simulink model "sma_corr"  (Simulink 8.0)
settings: Solver=VariableStepDiscrete  SolverMode=SingleTasking  StartTime=0.0  StopTime=1000  RelTol=1e-3
PreLoadFcn:
  | % Set this to 1 if simulating
  | simulating = 0;
  | 
  | % Overall parameters
  | n_comp = 128;
  | 
  | % Simulation
  | if (simulating)
  |     n_tvg_frames = 4;
  |     frames_per_vector = 4*8;
  |     vectors_per_wstep = 2;
  |     total_wsteps = 1; % 2^n
  |     % Laura's params
  |     x_ctr = 12;
  |     x_data_ctr = x_ctr-5;
  |     rx_addr_ctr = x_data_ctr+2;
  |     tx_addr_ctr = x_data_ctr+2;
  | else
  |     n_tvg_frames = 256;
  |     frames_per_vector = 256*8;
  |     vectors_per_wstep = 11;
  |     total_wsteps = 6; % 2^n
  |     % Laura's params
  |     x_ctr = 32;
  |     x_data_ctr = 18;
  |     rx_addr_ctr = x_data_ctr+2;
  |     tx_addr_ctr = x_data_ctr+2;
  | end
  | 
  | sb_states = repmat([ones(1, 256) zeros(1, 256)], 1, 2^(total_wsteps-1));
InitFcn:
  | % Get the XSG object to find the clock source
  | xps_xsg_blks = find_system(gcs,'FollowLinks','on','LookUnderMasks','all', 'SearchDepth',1, 'Tag','xps:xsg');
  | xsg_obj = xps_block(xps_xsg_blks{1}, {});
  | xsg_obj = xps_xsg(xsg_obj);
  | clk_src = get(xsg_obj, 'clk_src');
  | if strcmp(clk_src, 'adc0_clk')
  |     adc_brd = 'ZDOK 0';
  | elseif strcmp(clk_src, 'adc1_clk')
  |     adc_brd = 'ZDOK 1';
  | else
  |     adc_brd = '';
  | end
  | 
  | adc_clk_rate = 0;
  | % Get the appropriate ADC5g object
  | xps_adc5g_blks = find_system(gcs, 'FollowLinks', 'on', 'LookUnderMasks','all', 'SearchDepth',2, 'Tag','xps:adc5g');
  | for n = 1:length(xps_adc5g_blks)
  |     adc5g_blk = xps_adc5g_blks{n};
  |     if strcmp(get_param(adc5g_blk, 'adc_brd'), adc_brd)
  |         adc_clk_rate = 2*str2num(get_param(adc5g_blk, 'adc_clk_rate'));
  |     end
  | end
undefined symbols (not defined in the file or in its folder): Down, sfp, Up, X, xlipgetpartsetting, Z
libraries: xbsIndex_r4 x9231, casper_library_misc x178, dspsigops x32, casper_library_delays x26, xps_library x21
(part 24 of 92)

== system sma_corr/beng/sb1demodphase/patterns ==
  addr : Inport
  data_in : Inport  Port=2
  we : Inport  Port=3
  calc_add : Subsystem  mask{data_width=32, addr_width=10, sample_rate=sample_rate}
      mask prompts: data_width "data width"; addr_width "address width"; sample_rate "sample rate"
  convert_addr : xbsIndex_r4/Convert  gui_display_data_type=Fixed-point  arith_type=Unsigned  n_bits=addr_width  bin_pt=0  float_type=Single  exp_bits=8  fraction_bits=24  quantization=Truncate  overflow=Wrap  en=off  latency=0  dbl_ovrd=off  pipeline=off  block_type=convert
      settings: xl_area=[0, 0, 0, 0, 0, 0, 0]
  convert_din1 : xbsIndex_r4/Convert  gui_display_data_type=Fixed-point  arith_type=Unsigned  n_bits=32  bin_pt=0  float_type=Single  exp_bits=8  fraction_bits=24  quantization=Truncate  overflow=Wrap  en=off  latency=0  dbl_ovrd=off  pipeline=off  block_type=convert
      settings: xl_area=[0, 0, 0, 0, 0, 0, 0]
  convert_we : xbsIndex_r4/Convert  gui_display_data_type=Fixed-point  arith_type=Unsigned  n_bits=1  bin_pt=0  float_type=Single  exp_bits=8  fraction_bits=24  quantization=Truncate  overflow=Wrap  en=off  latency=0  dbl_ovrd=off  pipeline=off  block_type=convert
      settings: xl_area=[0, 0, 0, 0, 0, 0, 0]
  mem : Subsystem
  munge_in : Subsystem  AncestorBlock=casper_library_flow_control/munge  mask(munge){divisions=1, div_size=32, order=[0], arith_type_out=Unsigned, bin_pt_out=0}
      mask prompts: divisions "Number of divisions"; div_size "Division size (bits)"; order "Division packing order"; arith_type_out "Output arithmetic type"; bin_pt_out "Output binary point"
      mask help: Splits an input bus into the specified number of divisions and then recombines them in the order specified (most significant division = 0,least = number divisions-1)
      mask init:
      | munge_init(gcb, ...
      |     'divisions', divisions, ...
      |     'div_size', div_size, ...
      |     'order', order, ...
      |     'arith_type_out', arith_type_out, ...
      |     'bin_pt_out', bin_pt_out);
      settings: AttributesFormatString=|
      | split:32
      | join:0
      | Unsigned [32,0]
      mask TypeOptions arith_type_out: Unsigned | Signed  (2's comp) | Floating-point
  munge_out : Subsystem  AncestorBlock=casper_library_flow_control/munge  mask(munge){divisions=1, div_size=32, order=[0], arith_type_out=Unsigned, bin_pt_out=0}
      mask prompts: divisions "Number of divisions"; div_size "Division size (bits)"; order "Division packing order"; arith_type_out "Output arithmetic type"; bin_pt_out "Output binary point"
      mask help: Splits an input bus into the specified number of divisions and then recombines them in the order specified (most significant division = 0,least = number divisions-1)
      mask init:
      | munge_init(gcb, ...
      |     'divisions', divisions, ...
      |     'div_size', div_size, ...
      |     'order', order, ...
      |     'arith_type_out', arith_type_out, ...
      |     'bin_pt_out', bin_pt_out);
      settings: AttributesFormatString=|
      | split:32
      | join:0
      | Unsigned [32,0]
      mask TypeOptions arith_type_out: Unsigned | Signed  (2's comp) | Floating-point
  sma_corr_beng_sb1demodphase_patterns_addr : xbsIndex_r4/Gateway Out  inherit_from_input=off  hdl_port=on  timing_constraint=None  locs_specified=off  LOCs={'A5','B9','A9','C1','A3','G6','E9','A6','A7','A8','B8','B1','B3','D9','B5','B4','B6','C2'}  UseAsDAC=off  DACChannel='1'  block_type=gatewayout
      settings: infoedit="Gateway out block.  Converts Xilinx fixed point inputs into ouputs of type Simulink integer, double, or fixed point.<P><P>Hardware notes:  In hardware these blocks become top level output ports or are discarded, depending on how they are configured."  xl_area=[0, 0, 0, 0, 0, 0, 0]  sginterface={'Nallatech'=>{'xdspkit'=>{'non_memory_mapped_port'=>'uprev_Shared_BRAM_addr'}},'Xilinx'=>{'jtaghwcosim'=>{'non_memory_mapped_port'=>'uprev_Shared_BRAM_addr'},'xdspkit'=>{'non_memory_mapped_port'=>'uprev_Shared_BRAM_addr'}}}
  sma_corr_beng_sb1demodphase_patterns_data_in : xbsIndex_r4/Gateway Out  inherit_from_input=off  hdl_port=on  timing_constraint=None  locs_specified=off  LOCs={'B21','B22','A22','B23','B19','C19','A20','A21','A23','B24','A24','A25','E18','D18','C18','B18'}  UseAsDAC=off  DACChannel='1'  block_type=gatewayout
      settings: infoedit="Gateway out block.  Converts Xilinx fixed point inputs into ouputs of type Simulink integer, double, or fixed point.<P><P>Hardware notes:  In hardware these blocks become top level output ports or are discarded, depending on how they are configured."  xl_area=[0, 0, 0, 0, 0, 0, 0]  sginterface={'Nallatech'=>{'xdspkit'=>{'non_memory_mapped_port'=>'uprev_Shared_BRAM_data_in'}},'Xilinx'=>{'jtaghwcosim'=>{'non_memory_mapped_port'=>'uprev_Shared_BRAM_data_in'},'xdspkit'=>{'non_memory_mapped_port'=>'uprev_Shared_BRAM_data_in'}}}
  sma_corr_beng_sb1demodphase_patterns_data_out : xbsIndex_r4/Gateway In  gui_display_data_type=Fixed-point  arith_type=Unsigned  n_bits=32  bin_pt=0  preci_type=Single  exp_width=8  frac_width=24  quantization=Truncate  overflow=Wrap  period=sample_rate  dbl_ovrd=off  timing_constraint=None  locs_specified=off  LOCs={}  UseAsADC=off  ADCChannel='1'  inherit_from_input=off  hdl_port=on  block_type=gatewayin
      settings: xl_area=[0, 0, 0, 0, 0, 0, 0]
  sma_corr_beng_sb1demodphase_patterns_we : xbsIndex_r4/Gateway Out  inherit_from_input=off  hdl_port=on  timing_constraint=None  locs_specified=off  LOCs={'A14'}  UseAsDAC=off  DACChannel='1'  block_type=gatewayout
      settings: infoedit="Gateway out block.  Converts Xilinx fixed point inputs into ouputs of type Simulink integer, double, or fixed point.<P><P>Hardware notes:  In hardware these blocks become top level output ports or are discarded, depending on how they are configured."  xl_area=[0, 0, 0, 0, 0, 0, 0]
  data_out : Outport
  wires:
  convert_addr/1 -> sma_corr_beng_sb1demodphase_patterns_addr/1
  convert_din1/1 -> sma_corr_beng_sb1demodphase_patterns_data_in/1
  data_in/1 -> munge_in/1(din)
  calc_add/1(out) -> convert_addr/1
  sma_corr_beng_sb1demodphase_patterns_we/1 -> mem/3(In3)
  sma_corr_beng_sb1demodphase_patterns_data_in/1 -> mem/2(In2)
  sma_corr_beng_sb1demodphase_patterns_addr/1 -> mem/1(In1)
  convert_we/1 -> sma_corr_beng_sb1demodphase_patterns_we/1
  sma_corr_beng_sb1demodphase_patterns_data_out/1 -> munge_out/1(din)
  mem/1(Out1) -> sma_corr_beng_sb1demodphase_patterns_data_out/1
  addr/1 -> calc_add/1(in)
  we/1 -> convert_we/1
  munge_out/1(dout) -> data_out/1
  munge_in/1(dout) -> convert_din1/1

== system sma_corr/beng/sb1demodphase/pipeline ==
  d : Inport
  Register{0..4} (5 alike) : xbsIndex_r4/Register  init=0  rst=off  en=off  dbl_ovrd=off  block_type=register
      Register0 settings: block_version=11.4
      Register1 settings: block_version=11.4
      Register2 settings: block_version=11.4
  q : Outport
  wires:
  Register0/1 -> Register1/1
  Register1/1 -> Register2/1
  Register2/1 -> Register3/1
  Register3/1 -> Register4/1
  d/1 -> Register0/1
  Register4/1 -> q/1

== system sma_corr/beng/sb1demodphase/pipeline2 ==
  d : Inport
  Register{0..8} (9 alike) : xbsIndex_r4/Register  init=0  rst=off  en=off  dbl_ovrd=off  block_type=register
      Register0 settings: block_version=11.4
      Register1 settings: block_version=11.4
      Register2 settings: block_version=11.4
  q : Outport
  wires:
  Register0/1 -> Register1/1
  Register1/1 -> Register2/1
  Register2/1 -> Register3/1
  Register3/1 -> Register4/1
  Register4/1 -> Register5/1
  Register5/1 -> Register6/1
  Register6/1 -> Register7/1
  Register7/1 -> Register8/1
  d/1 -> Register0/1
  Register8/1 -> q/1

== system sma_corr/beng/sb1demodphase/pipeline3 ==
  d : Inport
  Register{0..8} (9 alike) : xbsIndex_r4/Register  init=0  rst=off  en=off  dbl_ovrd=off  block_type=register
      Register0 settings: block_version=11.4
      Register1 settings: block_version=11.4
      Register2 settings: block_version=11.4
  q : Outport
  wires:
  Register0/1 -> Register1/1
  Register1/1 -> Register2/1
  Register2/1 -> Register3/1
  Register3/1 -> Register4/1
  Register4/1 -> Register5/1
  Register5/1 -> Register6/1
  Register6/1 -> Register7/1
  Register7/1 -> Register8/1
  d/1 -> Register0/1
  Register8/1 -> q/1

== system sma_corr/beng/sb1demodphase/pipeline4 ==
  d : Inport
  Register{0..8} (9 alike) : xbsIndex_r4/Register  init=0  rst=off  en=off  dbl_ovrd=off  block_type=register
      Register0 settings: block_version=11.4
      Register1 settings: block_version=11.4
      Register2 settings: block_version=11.4
  q : Outport
  wires:
  Register0/1 -> Register1/1
  Register1/1 -> Register2/1
  Register2/1 -> Register3/1
  Register3/1 -> Register4/1
  Register4/1 -> Register5/1
  Register5/1 -> Register6/1
  Register6/1 -> Register7/1
  Register7/1 -> Register8/1
  d/1 -> Register0/1
  Register8/1 -> q/1

== system sma_corr/beng/sb1demodphase/pipeline5 ==
  d : Inport
  Register{0..4} (5 alike) : xbsIndex_r4/Register  init=0  rst=off  en=off  dbl_ovrd=off  block_type=register
      Register0 settings: block_version=11.4
      Register1 settings: block_version=11.4
      Register2 settings: block_version=11.4
  q : Outport
  wires:
  Register0/1 -> Register1/1
  Register1/1 -> Register2/1
  Register2/1 -> Register3/1
  Register3/1 -> Register4/1
  d/1 -> Register0/1
  Register4/1 -> q/1

== system sma_corr/beng/sb1demodphase/pipeline6 ==
  d : Inport
  Register{0..4} (5 alike) : xbsIndex_r4/Register  init=0  rst=off  en=off  dbl_ovrd=off  block_type=register
      Register0 settings: block_version=11.4
      Register1 settings: block_version=11.4
      Register2 settings: block_version=11.4
  q : Outport
  wires:
  Register0/1 -> Register1/1
  Register1/1 -> Register2/1
  Register2/1 -> Register3/1
  Register3/1 -> Register4/1
  d/1 -> Register0/1
  Register4/1 -> q/1

== system sma_corr/beng/sb1demodphase/pipeline7 ==
  d : Inport
  Register{0..4} (5 alike) : xbsIndex_r4/Register  init=0  rst=off  en=off  dbl_ovrd=off  block_type=register
      Register0 settings: block_version=11.4
      Register1 settings: block_version=11.4
      Register2 settings: block_version=11.4
  q : Outport
  wires:
  Register0/1 -> Register1/1
  Register1/1 -> Register2/1
  Register2/1 -> Register3/1
  Register3/1 -> Register4/1
  d/1 -> Register0/1
  Register4/1 -> q/1

== system sma_corr/beng/sb1demodphase/pipeline8 ==
  d : Inport
  Register{0..4} (5 alike) : xbsIndex_r4/Register  init=0  rst=off  en=off  dbl_ovrd=off  block_type=register
      Register0 settings: block_version=11.4
      Register1 settings: block_version=11.4
      Register2 settings: block_version=11.4
  q : Outport
  wires:
  Register0/1 -> Register1/1
  Register1/1 -> Register2/1
  Register2/1 -> Register3/1
  Register3/1 -> Register4/1
  d/1 -> Register0/1
  Register4/1 -> q/1

== system sma_corr/beng/sb1demodphase/scramble ==
  note: Each input a single polarization. This block rearranges data to interleave
      | the polarizations in each stream to match the expected input of subsequent
      | systems.
  X : Inport
  Y : Inport  Port=2
  sync : Inport  Port=3
  BitBasher : xbsIndex_r4/BitBasher  bitexpr=|  display_expr=off  sr_1=1  arith_type1=Unsigned  bin_pt1=0  sr_2=2  arith_type2=Unsigned  bin_pt2=0  sr_3=3  arith_type3=Unsigned  bin_pt3=0  sr_4=4  arith_type4=Unsigned  bin_pt4=0  dbl_ovrd=off  block_type=bitbasher
      | <!DOCTYPE HTML PUBLIC "-//W3C//DTD HTML 4.0//EN" "http://www.w3.org/TR/REC-html40/strict.dtd">
      | <html><head><meta name="qrichtext" content="1" /><style type="text/css">
      | p, li { white-space: pre-wrap; }
      | </style></head><body style=" font-family:'Ubuntu'; font-size:11pt; font-weight:400; font-style:normal;">
      | <p style=" margin-top:0px; margin-bottom:0px; margin-left:0px; margin-right:0px; -qt-block-indent:0; text-indent:0px;"><span style=" font-family:'MS Shell Dlg'; font-size:8pt;">Xab={X[127:96]}<br />Xcd={X[95:64]}<br />Xef={X[63:32]}<br />Xgh={X[31:0]}</span></p></body></html>
  BitBasher1 : xbsIndex_r4/BitBasher  bitexpr=|  display_expr=off  sr_1=1  arith_type1=Unsigned  bin_pt1=0  sr_2=2  arith_type2=Unsigned  bin_pt2=0  sr_3=3  arith_type3=Unsigned  bin_pt3=0  sr_4=4  arith_type4=Unsigned  bin_pt4=0  dbl_ovrd=off  block_type=bitbasher
      | <!DOCTYPE HTML PUBLIC "-//W3C//DTD HTML 4.0//EN" "http://www.w3.org/TR/REC-html40/strict.dtd">
      | <html><head><meta name="qrichtext" content="1" /><style type="text/css">
      | p, li { white-space: pre-wrap; }
      | </style></head><body style=" font-family:'Ubuntu'; font-size:11pt; font-weight:400; font-style:normal;">
      | <p style=" margin-top:0px; margin-bottom:0px; margin-left:0px; margin-right:0px; -qt-block-indent:0; text-indent:0px;"><span style=" font-family:'MS Shell Dlg'; font-size:8pt;">Yab={Y[127:96]}<br />Ycd={Y[95:64]}<br />Yef={Y[63:32]}<br />Ygh={Y[31:0]}</span></p></body></html>
  BitBasher2 : xbsIndex_r4/BitBasher  bitexpr=|  display_expr=off  sr_1=1  arith_type1=Unsigned  bin_pt1=0  sr_2=2  arith_type2=Unsigned  bin_pt2=0  sr_3=3  arith_type3=Unsigned  bin_pt3=0  sr_4=4  arith_type4=Unsigned  bin_pt4=0  dbl_ovrd=off  block_type=bitbasher
      | <!DOCTYPE HTML PUBLIC "-//W3C//DTD HTML 4.0//EN" "http://www.w3.org/TR/REC-html40/strict.dtd">
      | <html><head><meta name="qrichtext" content="1" /><style type="text/css">
      | p, li { white-space: pre-wrap; }
      | </style></head><body style=" font-family:'Ubuntu'; font-size:11pt; font-weight:400; font-style:normal;">
      | <p style=" margin-top:0px; margin-bottom:0px; margin-left:0px; margin-right:0px; -qt-block-indent:0; text-indent:0px;"><span style=" font-family:'MS Shell Dlg'; font-size:8pt;">d0={Xab[31:16],Yab[31:16],Xab[15:0],Yab[15:0],Xcd[31:16],Ycd[31:16],Xcd[15:0],Ycd[15:0]}</span></p></body></html>
  BitBasher3 : xbsIndex_r4/BitBasher  bitexpr=|  display_expr=off  sr_1=1  arith_type1=Unsigned  bin_pt1=0  sr_2=2  arith_type2=Unsigned  bin_pt2=0  sr_3=3  arith_type3=Unsigned  bin_pt3=0  sr_4=4  arith_type4=Unsigned  bin_pt4=0  dbl_ovrd=off  block_type=bitbasher
      | <!DOCTYPE HTML PUBLIC "-//W3C//DTD HTML 4.0//EN" "http://www.w3.org/TR/REC-html40/strict.dtd">
      | <html><head><meta name="qrichtext" content="1" /><style type="text/css">
      | p, li { white-space: pre-wrap; }
      | </style></head><body style=" font-family:'Ubuntu'; font-size:11pt; font-weight:400; font-style:normal;">
      | <p style=" margin-top:0px; margin-bottom:0px; margin-left:0px; margin-right:0px; -qt-block-indent:0; text-indent:0px;"><span style=" font-family:'MS Shell Dlg'; font-size:8pt;">d1={Xef[31:16],Yef[31:16],Xef[15:0],Yef[15:0],Xgh[31:16],Ygh[31:16],Xgh[15:0],Ygh[15:0]}</span></p></body></html>
  d0 : Outport
  d1 : Outport  Port=2
  sync_out : Outport  Port=3
  wires:
  BitBasher3/1 -> d1/1
  BitBasher2/1 -> d0/1
  X/1 -> BitBasher/1
  Y/1 -> BitBasher1/1
  sync/1 -> sync_out/1
  BitBasher/1 -> BitBasher2/1
  BitBasher/2 -> BitBasher2/2
  BitBasher1/1 -> BitBasher2/3
  BitBasher1/2 -> BitBasher2/4
  BitBasher/3 -> BitBasher3/1
  BitBasher/4 -> BitBasher3/2
  BitBasher1/3 -> BitBasher3/3
  BitBasher1/4 -> BitBasher3/4

== system sma_corr/beng/sb1demodphase/unscramble ==
  note: Each input contains 4+4-bit complex for pol0 and pol1 interleaved. This block
      | rearranges the data so that each polarization is in a single stream.
  d0 : Inport
  d1 : Inport  Port=2
  BitBasher : xbsIndex_r4/BitBasher  bitexpr=|  display_expr=off  sr_1=1  arith_type1=Unsigned  bin_pt1=0  sr_2=2  arith_type2=Unsigned  bin_pt2=0  sr_3=3  arith_type3=Unsigned  bin_pt3=0  sr_4=4  arith_type4=Unsigned  bin_pt4=0  dbl_ovrd=off  block_type=bitbasher
      | <!DOCTYPE HTML PUBLIC "-//W3C//DTD HTML 4.0//EN" "http://www.w3.org/TR/REC-html40/strict.dtd">
      | <html><head><meta name="qrichtext" content="1" /><style type="text/css">
      | p, li { white-space: pre-wrap; }
      | </style></head><body style=" font-family:'Ubuntu'; font-size:11pt; font-weight:400; font-style:normal;">
      | <p style=" margin-top:0px; margin-bottom:0px; margin-left:0px; margin-right:0px; -qt-block-indent:0; text-indent:0px;"><span style=" font-family:'MS Shell Dlg'; font-size:8pt;">Xabcd={i[63:56], i[47:40], i[31:24], i[15:8]}<br />Yabcd={i[55:48], i[39:32], i[23:16], i[7:0]}<br /></span></p></body></html>
  BitBasher1 : xbsIndex_r4/BitBasher  bitexpr=|  display_expr=off  sr_1=1  arith_type1=Unsigned  bin_pt1=0  sr_2=2  arith_type2=Unsigned  bin_pt2=0  sr_3=3  arith_type3=Unsigned  bin_pt3=0  sr_4=4  arith_type4=Unsigned  bin_pt4=0  dbl_ovrd=off  block_type=bitbasher
      | <!DOCTYPE HTML PUBLIC "-//W3C//DTD HTML 4.0//EN" "http://www.w3.org/TR/REC-html40/strict.dtd">
      | <html><head><meta name="qrichtext" content="1" /><style type="text/css">
      | p, li { white-space: pre-wrap; }
      | </style></head><body style=" font-family:'Ubuntu'; font-size:11pt; font-weight:400; font-style:normal;">
      | <p style=" margin-top:0px; margin-bottom:0px; margin-left:0px; margin-right:0px; -qt-block-indent:0; text-indent:0px;"><span style=" font-family:'MS Shell Dlg'; font-size:8pt;">Xefgh={i[63:56], i[47:40], i[31:24], i[15:8]}<br />Yefgh={i[55:48], i[39:32], i[23:16], i[7:0]}<br /></span></p></body></html>
  BitBasher2 : xbsIndex_r4/BitBasher  bitexpr=|  display_expr=off  sr_1=1  arith_type1=Unsigned  bin_pt1=0  sr_2=2  arith_type2=Unsigned  bin_pt2=0  sr_3=3  arith_type3=Unsigned  bin_pt3=0  sr_4=4  arith_type4=Unsigned  bin_pt4=0  dbl_ovrd=off  block_type=bitbasher
      | <!DOCTYPE HTML PUBLIC "-//W3C//DTD HTML 4.0//EN" "http://www.w3.org/TR/REC-html40/strict.dtd">
      | <html><head><meta name="qrichtext" content="1" /><style type="text/css">
      | p, li { white-space: pre-wrap; }
      | </style></head><body style=" font-family:'Ubuntu'; font-size:11pt; font-weight:400; font-style:normal;">
      | <p style=" margin-top:12px; margin-bottom:12px; margin-left:0px; margin-right:0px; -qt-block-indent:0; text-indent:0px;"><span style=" font-family:'MS Shell Dlg'; font-size:8pt;">X={Xabcd,Xefgh}</span></p></body></html>
  BitBasher3 : xbsIndex_r4/BitBasher  bitexpr=|  display_expr=off  sr_1=1  arith_type1=Unsigned  bin_pt1=0  sr_2=2  arith_type2=Unsigned  bin_pt2=0  sr_3=3  arith_type3=Unsigned  bin_pt3=0  sr_4=4  arith_type4=Unsigned  bin_pt4=0  dbl_ovrd=off  block_type=bitbasher
      | <!DOCTYPE HTML PUBLIC "-//W3C//DTD HTML 4.0//EN" "http://www.w3.org/TR/REC-html40/strict.dtd">
      | <html><head><meta name="qrichtext" content="1" /><style type="text/css">
      | p, li { white-space: pre-wrap; }
      | </style></head><body style=" font-family:'Ubuntu'; font-size:11pt; font-weight:400; font-style:normal;">
      | <p style=" margin-top:12px; margin-bottom:12px; margin-left:0px; margin-right:0px; -qt-block-indent:0; text-indent:0px;"><span style=" font-family:'MS Shell Dlg'; font-size:8pt;">Y={Yabcd,Yefgh}</span></p></body></html>
  x : Outport
  y : Outport  Port=2
  wires:
  d0/1 -> BitBasher/1
  BitBasher2/1 -> x/1
  BitBasher3/1 -> y/1
  d1/1 -> BitBasher1/1
  BitBasher1/1 -> BitBasher2/2
  BitBasher/1 -> BitBasher2/1
  BitBasher/2 -> BitBasher3/1
  BitBasher1/2 -> BitBasher3/2

== system sma_corr/beng/sum0/sum_Xab ==
  gain : Inport
  sync_in : Inport  Port=2
  in16 : Inport  Port=3
  disable : Inport  Port=4
  BitBasher2 : xbsIndex_r4/BitBasher  bitexpr=|  display_expr=off  sr_1=1  arith_type1=Unsigned  bin_pt1=0  sr_2=2  arith_type2=Unsigned  bin_pt2=0  sr_3=3  arith_type3=Unsigned  bin_pt3=0  sr_4=4  arith_type4=Unsigned  bin_pt4=0  dbl_ovrd=off  block_type=bitbasher
      | <!DOCTYPE HTML PUBLIC "-//W3C//DTD HTML 4.0//EN" "http://www.w3.org/TR/REC-html40/strict.dtd">
      | <html><head><meta name="qrichtext" content="1" /><style type="text/css">
      | p, li { white-space: pre-wrap; }
      | </style></head><body style=" font-family:'Sans'; font-size:10pt; font-weight:400; font-style:normal;">
      | <p style=" margin-top:12px; margin-bottom:12px; margin-left:0px; margin-right:0px; -qt-block-indent:0; text-indent:0px;"><span style=" font-family:'MS Shell Dlg'; font-size:8pt;">o={w,x,y,z}</span></p></body></html>
  Delay : xbsIndex_r4/Delay  rst=off  infoeditControl=Selection of Reset will increase slice count due to use of real FFs and instead of SRLs  en=off  latency=7  dbl_ovrd=off  reg_retiming=off  block_type=delay
  Delay1 : xbsIndex_r4/Delay  rst=off  infoeditControl=Selection of Reset will increase slice count due to use of real FFs and instead of SRLs  en=off  latency=7  dbl_ovrd=off  reg_retiming=off  block_type=delay
  gain_and_quantize : Subsystem
  gain_and_quantize1 : Subsystem
  gain_and_quantize2 : Subsystem
  gain_and_quantize3 : Subsystem
      settings: AttributesFormatString=latency = 7
  simd_vacc : Subsystem
  sync_out : Outport
  valid : Outport  Port=2
  sum16 : Outport  Port=3
  wires:
  gain_and_quantize/1(out4) -> BitBasher2/1
  gain/1 -> gain_and_quantize/1(g), gain_and_quantize1/1(g), gain_and_quantize2/1(g), gain_and_quantize3/1(g)
  gain_and_quantize1/1(out4) -> BitBasher2/2
  simd_vacc/3(w) -> gain_and_quantize/2(in8)
  gain_and_quantize2/1(out4) -> BitBasher2/3
  simd_vacc/6(z) -> gain_and_quantize3/2(in8)
  sync_in/1 -> simd_vacc/1(sync)
  in16/1 -> simd_vacc/2(in)
  simd_vacc/4(x) -> gain_and_quantize1/2(in8)
  simd_vacc/5(y) -> gain_and_quantize2/2(in8)
  simd_vacc/1(sync_out) -> Delay/1
  simd_vacc/2(valid) -> Delay1/1
  BitBasher2/1 -> sum16/1
  gain_and_quantize3/1(out4) -> BitBasher2/4
  Delay1/1 -> valid/1
  Delay/1 -> sync_out/1
  disable/1 -> simd_vacc/3(disable)

== system sma_corr/beng/sum0/sum_Xcd ==
  gain : Inport
  sync_in : Inport  Port=2
  in16 : Inport  Port=3
  disable : Inport  Port=4
  BitBasher2 : xbsIndex_r4/BitBasher  bitexpr=|  display_expr=off  sr_1=1  arith_type1=Unsigned  bin_pt1=0  sr_2=2  arith_type2=Unsigned  bin_pt2=0  sr_3=3  arith_type3=Unsigned  bin_pt3=0  sr_4=4  arith_type4=Unsigned  bin_pt4=0  dbl_ovrd=off  block_type=bitbasher
      | <!DOCTYPE HTML PUBLIC "-//W3C//DTD HTML 4.0//EN" "http://www.w3.org/TR/REC-html40/strict.dtd">
      | <html><head><meta name="qrichtext" content="1" /><style type="text/css">
      | p, li { white-space: pre-wrap; }
      | </style></head><body style=" font-family:'Sans'; font-size:10pt; font-weight:400; font-style:normal;">
      | <p style=" margin-top:12px; margin-bottom:12px; margin-left:0px; margin-right:0px; -qt-block-indent:0; text-indent:0px;"><span style=" font-family:'MS Shell Dlg'; font-size:8pt;">o={w,x,y,z}</span></p></body></html>
  Terminator : Terminator
  Terminator1 : Terminator
  gain_and_quantize : Subsystem
  gain_and_quantize1 : Subsystem
  gain_and_quantize2 : Subsystem
  gain_and_quantize3 : Subsystem
      settings: AttributesFormatString=latency = 7
  simd_vacc : Subsystem
  sum16 : Outport
  wires:
  gain_and_quantize/1(out4) -> BitBasher2/1
  gain/1 -> gain_and_quantize/1(g), gain_and_quantize1/1(g), gain_and_quantize2/1(g), gain_and_quantize3/1(g)
  gain_and_quantize1/1(out4) -> BitBasher2/2
  simd_vacc/3(w) -> gain_and_quantize/2(in8)
  gain_and_quantize2/1(out4) -> BitBasher2/3
  simd_vacc/6(z) -> gain_and_quantize3/2(in8)
  sync_in/1 -> simd_vacc/1(sync)
  in16/1 -> simd_vacc/2(in)
  simd_vacc/4(x) -> gain_and_quantize1/2(in8)
  simd_vacc/5(y) -> gain_and_quantize2/2(in8)
  BitBasher2/1 -> sum16/1
  gain_and_quantize3/1(out4) -> BitBasher2/4
  disable/1 -> simd_vacc/3(disable)
  simd_vacc/1(sync_out) -> Terminator/1
  simd_vacc/2(valid) -> Terminator1/1

== system sma_corr/beng/sum0/sum_Yab ==
  gain : Inport
  sync_in : Inport  Port=2
  in16 : Inport  Port=3
  disable : Inport  Port=4
  BitBasher2 : xbsIndex_r4/BitBasher  bitexpr=|  display_expr=off  sr_1=1  arith_type1=Unsigned  bin_pt1=0  sr_2=2  arith_type2=Unsigned  bin_pt2=0  sr_3=3  arith_type3=Unsigned  bin_pt3=0  sr_4=4  arith_type4=Unsigned  bin_pt4=0  dbl_ovrd=off  block_type=bitbasher
      | <!DOCTYPE HTML PUBLIC "-//W3C//DTD HTML 4.0//EN" "http://www.w3.org/TR/REC-html40/strict.dtd">
      | <html><head><meta name="qrichtext" content="1" /><style type="text/css">
      | p, li { white-space: pre-wrap; }
      | </style></head><body style=" font-family:'Sans'; font-size:10pt; font-weight:400; font-style:normal;">
      | <p style=" margin-top:12px; margin-bottom:12px; margin-left:0px; margin-right:0px; -qt-block-indent:0; text-indent:0px;"><span style=" font-family:'MS Shell Dlg'; font-size:8pt;">o={w,x,y,z}</span></p></body></html>
  Terminator : Terminator
  Terminator1 : Terminator
  gain_and_quantize : Subsystem
  gain_and_quantize1 : Subsystem
  gain_and_quantize2 : Subsystem
  gain_and_quantize3 : Subsystem
      settings: AttributesFormatString=latency = 7
  simd_vacc : Subsystem
  sum16 : Outport
  wires:
  simd_vacc/2(valid) -> Terminator1/1
  simd_vacc/1(sync_out) -> Terminator/1
  disable/1 -> simd_vacc/3(disable)
  gain_and_quantize3/1(out4) -> BitBasher2/4
  BitBasher2/1 -> sum16/1
  simd_vacc/5(y) -> gain_and_quantize2/2(in8)
  simd_vacc/4(x) -> gain_and_quantize1/2(in8)
  in16/1 -> simd_vacc/2(in)
  sync_in/1 -> simd_vacc/1(sync)
  simd_vacc/6(z) -> gain_and_quantize3/2(in8)
  gain_and_quantize2/1(out4) -> BitBasher2/3
  simd_vacc/3(w) -> gain_and_quantize/2(in8)
  gain_and_quantize1/1(out4) -> BitBasher2/2
  gain/1 -> gain_and_quantize3/1(g), gain_and_quantize2/1(g), gain_and_quantize1/1(g), gain_and_quantize/1(g)
  gain_and_quantize/1(out4) -> BitBasher2/1

== system sma_corr/beng/sum0/sum_Ycd ==
  gain : Inport
  sync_in : Inport  Port=2
  in16 : Inport  Port=3
  disable : Inport  Port=4
  BitBasher2 : xbsIndex_r4/BitBasher  bitexpr=|  display_expr=off  sr_1=1  arith_type1=Unsigned  bin_pt1=0  sr_2=2  arith_type2=Unsigned  bin_pt2=0  sr_3=3  arith_type3=Unsigned  bin_pt3=0  sr_4=4  arith_type4=Unsigned  bin_pt4=0  dbl_ovrd=off  block_type=bitbasher
      | <!DOCTYPE HTML PUBLIC "-//W3C//DTD HTML 4.0//EN" "http://www.w3.org/TR/REC-html40/strict.dtd">
      | <html><head><meta name="qrichtext" content="1" /><style type="text/css">
      | p, li { white-space: pre-wrap; }
      | </style></head><body style=" font-family:'Sans'; font-size:10pt; font-weight:400; font-style:normal;">
      | <p style=" margin-top:12px; margin-bottom:12px; margin-left:0px; margin-right:0px; -qt-block-indent:0; text-indent:0px;"><span style=" font-family:'MS Shell Dlg'; font-size:8pt;">o={w,x,y,z}</span></p></body></html>
  Terminator : Terminator
  Terminator1 : Terminator
  gain_and_quantize : Subsystem
  gain_and_quantize1 : Subsystem
  gain_and_quantize2 : Subsystem
  gain_and_quantize3 : Subsystem
      settings: AttributesFormatString=latency = 7
  simd_vacc : Subsystem
  sum16 : Outport
  wires:
  gain_and_quantize/1(out4) -> BitBasher2/1
  gain/1 -> gain_and_quantize/1(g), gain_and_quantize1/1(g), gain_and_quantize2/1(g), gain_and_quantize3/1(g)
  gain_and_quantize1/1(out4) -> BitBasher2/2
  simd_vacc/3(w) -> gain_and_quantize/2(in8)
  gain_and_quantize2/1(out4) -> BitBasher2/3
  simd_vacc/6(z) -> gain_and_quantize3/2(in8)
  sync_in/1 -> simd_vacc/1(sync)
  in16/1 -> simd_vacc/2(in)
  simd_vacc/4(x) -> gain_and_quantize1/2(in8)
  simd_vacc/5(y) -> gain_and_quantize2/2(in8)
  BitBasher2/1 -> sum16/1
  gain_and_quantize3/1(out4) -> BitBasher2/4
  disable/1 -> simd_vacc/3(disable)
  simd_vacc/1(sync_out) -> Terminator/1
  simd_vacc/2(valid) -> Terminator1/1

== system sma_corr/beng/sum1/sum_Xab ==
  gain : Inport
  sync_in : Inport  Port=2
  in16 : Inport  Port=3
  disable : Inport  Port=4
  BitBasher2 : xbsIndex_r4/BitBasher  bitexpr=|  display_expr=off  sr_1=1  arith_type1=Unsigned  bin_pt1=0  sr_2=2  arith_type2=Unsigned  bin_pt2=0  sr_3=3  arith_type3=Unsigned  bin_pt3=0  sr_4=4  arith_type4=Unsigned  bin_pt4=0  dbl_ovrd=off  block_type=bitbasher
      | <!DOCTYPE HTML PUBLIC "-//W3C//DTD HTML 4.0//EN" "http://www.w3.org/TR/REC-html40/strict.dtd">
      | <html><head><meta name="qrichtext" content="1" /><style type="text/css">
      | p, li { white-space: pre-wrap; }
      | </style></head><body style=" font-family:'Sans'; font-size:10pt; font-weight:400; font-style:normal;">
      | <p style=" margin-top:12px; margin-bottom:12px; margin-left:0px; margin-right:0px; -qt-block-indent:0; text-indent:0px;"><span style=" font-family:'MS Shell Dlg'; font-size:8pt;">o={w,x,y,z}</span></p></body></html>
  Delay : xbsIndex_r4/Delay  rst=off  infoeditControl=Selection of Reset will increase slice count due to use of real FFs and instead of SRLs  en=off  latency=7  dbl_ovrd=off  reg_retiming=off  block_type=delay
  Delay1 : xbsIndex_r4/Delay  rst=off  infoeditControl=Selection of Reset will increase slice count due to use of real FFs and instead of SRLs  en=off  latency=7  dbl_ovrd=off  reg_retiming=off  block_type=delay
  gain_and_quantize : Subsystem
  gain_and_quantize1 : Subsystem
  gain_and_quantize2 : Subsystem
  gain_and_quantize3 : Subsystem
      settings: AttributesFormatString=latency = 7
  simd_vacc : Subsystem
  sync_out : Outport
  valid : Outport  Port=2
  sum16 : Outport  Port=3
  wires:
  gain_and_quantize/1(out4) -> BitBasher2/1
  gain/1 -> gain_and_quantize/1(g), gain_and_quantize1/1(g), gain_and_quantize2/1(g), gain_and_quantize3/1(g)
  gain_and_quantize1/1(out4) -> BitBasher2/2
  simd_vacc/3(w) -> gain_and_quantize/2(in8)
  gain_and_quantize2/1(out4) -> BitBasher2/3
  simd_vacc/6(z) -> gain_and_quantize3/2(in8)
  sync_in/1 -> simd_vacc/1(sync)
  in16/1 -> simd_vacc/2(in)
  simd_vacc/4(x) -> gain_and_quantize1/2(in8)
  simd_vacc/5(y) -> gain_and_quantize2/2(in8)
  simd_vacc/1(sync_out) -> Delay/1
  simd_vacc/2(valid) -> Delay1/1
  BitBasher2/1 -> sum16/1
  gain_and_quantize3/1(out4) -> BitBasher2/4
  Delay1/1 -> valid/1
  Delay/1 -> sync_out/1
  disable/1 -> simd_vacc/3(disable)

== system sma_corr/beng/sum1/sum_Xcd ==
  gain : Inport
  sync_in : Inport  Port=2
  in16 : Inport  Port=3
  disable : Inport  Port=4
  BitBasher2 : xbsIndex_r4/BitBasher  bitexpr=|  display_expr=off  sr_1=1  arith_type1=Unsigned  bin_pt1=0  sr_2=2  arith_type2=Unsigned  bin_pt2=0  sr_3=3  arith_type3=Unsigned  bin_pt3=0  sr_4=4  arith_type4=Unsigned  bin_pt4=0  dbl_ovrd=off  block_type=bitbasher
      | <!DOCTYPE HTML PUBLIC "-//W3C//DTD HTML 4.0//EN" "http://www.w3.org/TR/REC-html40/strict.dtd">
      | <html><head><meta name="qrichtext" content="1" /><style type="text/css">
      | p, li { white-space: pre-wrap; }
      | </style></head><body style=" font-family:'Sans'; font-size:10pt; font-weight:400; font-style:normal;">
      | <p style=" margin-top:12px; margin-bottom:12px; margin-left:0px; margin-right:0px; -qt-block-indent:0; text-indent:0px;"><span style=" font-family:'MS Shell Dlg'; font-size:8pt;">o={w,x,y,z}</span></p></body></html>
  Terminator : Terminator
  Terminator1 : Terminator
  gain_and_quantize : Subsystem
  gain_and_quantize1 : Subsystem
  gain_and_quantize2 : Subsystem
  gain_and_quantize3 : Subsystem
      settings: AttributesFormatString=latency = 7
  simd_vacc : Subsystem
  sum16 : Outport
  wires:
  gain_and_quantize/1(out4) -> BitBasher2/1
  gain/1 -> gain_and_quantize/1(g), gain_and_quantize1/1(g), gain_and_quantize2/1(g), gain_and_quantize3/1(g)
  gain_and_quantize1/1(out4) -> BitBasher2/2
  simd_vacc/3(w) -> gain_and_quantize/2(in8)
  gain_and_quantize2/1(out4) -> BitBasher2/3
  simd_vacc/6(z) -> gain_and_quantize3/2(in8)
  sync_in/1 -> simd_vacc/1(sync)
  in16/1 -> simd_vacc/2(in)
  simd_vacc/4(x) -> gain_and_quantize1/2(in8)
  simd_vacc/5(y) -> gain_and_quantize2/2(in8)
  BitBasher2/1 -> sum16/1
  gain_and_quantize3/1(out4) -> BitBasher2/4
  disable/1 -> simd_vacc/3(disable)
  simd_vacc/1(sync_out) -> Terminator/1
  simd_vacc/2(valid) -> Terminator1/1

== system sma_corr/beng/sum1/sum_Yab ==
  gain : Inport
  sync_in : Inport  Port=2
  in16 : Inport  Port=3
  disable : Inport  Port=4
  BitBasher2 : xbsIndex_r4/BitBasher  bitexpr=|  display_expr=off  sr_1=1  arith_type1=Unsigned  bin_pt1=0  sr_2=2  arith_type2=Unsigned  bin_pt2=0  sr_3=3  arith_type3=Unsigned  bin_pt3=0  sr_4=4  arith_type4=Unsigned  bin_pt4=0  dbl_ovrd=off  block_type=bitbasher
      | <!DOCTYPE HTML PUBLIC "-//W3C//DTD HTML 4.0//EN" "http://www.w3.org/TR/REC-html40/strict.dtd">
      | <html><head><meta name="qrichtext" content="1" /><style type="text/css">
      | p, li { white-space: pre-wrap; }
      | </style></head><body style=" font-family:'Sans'; font-size:10pt; font-weight:400; font-style:normal;">
      | <p style=" margin-top:12px; margin-bottom:12px; margin-left:0px; margin-right:0px; -qt-block-indent:0; text-indent:0px;"><span style=" font-family:'MS Shell Dlg'; font-size:8pt;">o={w,x,y,z}</span></p></body></html>
  Terminator : Terminator
  Terminator1 : Terminator
  gain_and_quantize : Subsystem
  gain_and_quantize1 : Subsystem
  gain_and_quantize2 : Subsystem
  gain_and_quantize3 : Subsystem
      settings: AttributesFormatString=latency = 7
  simd_vacc : Subsystem
  sum16 : Outport
  wires:
  simd_vacc/2(valid) -> Terminator1/1
  simd_vacc/1(sync_out) -> Terminator/1
  disable/1 -> simd_vacc/3(disable)
  gain_and_quantize3/1(out4) -> BitBasher2/4
  BitBasher2/1 -> sum16/1
  simd_vacc/5(y) -> gain_and_quantize2/2(in8)
  simd_vacc/4(x) -> gain_and_quantize1/2(in8)
  in16/1 -> simd_vacc/2(in)
  sync_in/1 -> simd_vacc/1(sync)
  simd_vacc/6(z) -> gain_and_quantize3/2(in8)
  gain_and_quantize2/1(out4) -> BitBasher2/3
  simd_vacc/3(w) -> gain_and_quantize/2(in8)
  gain_and_quantize1/1(out4) -> BitBasher2/2
  gain/1 -> gain_and_quantize3/1(g), gain_and_quantize2/1(g), gain_and_quantize1/1(g), gain_and_quantize/1(g)
  gain_and_quantize/1(out4) -> BitBasher2/1

== system sma_corr/beng/sum1/sum_Ycd ==
  gain : Inport
  sync_in : Inport  Port=2
  in16 : Inport  Port=3
  disable : Inport  Port=4
  BitBasher2 : xbsIndex_r4/BitBasher  bitexpr=|  display_expr=off  sr_1=1  arith_type1=Unsigned  bin_pt1=0  sr_2=2  arith_type2=Unsigned  bin_pt2=0  sr_3=3  arith_type3=Unsigned  bin_pt3=0  sr_4=4  arith_type4=Unsigned  bin_pt4=0  dbl_ovrd=off  block_type=bitbasher
      | <!DOCTYPE HTML PUBLIC "-//W3C//DTD HTML 4.0//EN" "http://www.w3.org/TR/REC-html40/strict.dtd">
      | <html><head><meta name="qrichtext" content="1" /><style type="text/css">
      | p, li { white-space: pre-wrap; }
      | </style></head><body style=" font-family:'Sans'; font-size:10pt; font-weight:400; font-style:normal;">
      | <p style=" margin-top:12px; margin-bottom:12px; margin-left:0px; margin-right:0px; -qt-block-indent:0; text-indent:0px;"><span style=" font-family:'MS Shell Dlg'; font-size:8pt;">o={w,x,y,z}</span></p></body></html>
  Terminator : Terminator
  Terminator1 : Terminator
  gain_and_quantize : Subsystem
  gain_and_quantize1 : Subsystem
  gain_and_quantize2 : Subsystem
  gain_and_quantize3 : Subsystem
      settings: AttributesFormatString=latency = 7
  simd_vacc : Subsystem
  sum16 : Outport
  wires:
  gain_and_quantize/1(out4) -> BitBasher2/1
  gain/1 -> gain_and_quantize/1(g), gain_and_quantize1/1(g), gain_and_quantize2/1(g), gain_and_quantize3/1(g)
  gain_and_quantize1/1(out4) -> BitBasher2/2
  simd_vacc/3(w) -> gain_and_quantize/2(in8)
  gain_and_quantize2/1(out4) -> BitBasher2/3
  simd_vacc/6(z) -> gain_and_quantize3/2(in8)
  sync_in/1 -> simd_vacc/1(sync)
  in16/1 -> simd_vacc/2(in)
  simd_vacc/4(x) -> gain_and_quantize1/2(in8)
  simd_vacc/5(y) -> gain_and_quantize2/2(in8)
  BitBasher2/1 -> sum16/1
  gain_and_quantize3/1(out4) -> BitBasher2/4
  disable/1 -> simd_vacc/3(disable)
  simd_vacc/1(sync_out) -> Terminator/1
  simd_vacc/2(valid) -> Terminator1/1

== system sma_corr/beng/sum2/sum_Xab ==
  gain : Inport
  sync_in : Inport  Port=2
  in32 : Inport  Port=3
  disable : Inport  Port=4
  BitBasher2 : xbsIndex_r4/BitBasher  bitexpr=|  display_expr=off  sr_1=1  arith_type1=Unsigned  bin_pt1=0  sr_2=2  arith_type2=Unsigned  bin_pt2=0  sr_3=3  arith_type3=Unsigned  bin_pt3=0  sr_4=4  arith_type4=Unsigned  bin_pt4=0  dbl_ovrd=off  block_type=bitbasher
      | <!DOCTYPE HTML PUBLIC "-//W3C//DTD HTML 4.0//EN" "http://www.w3.org/TR/REC-html40/strict.dtd">
      | <html><head><meta name="qrichtext" content="1" /><style type="text/css">
      | p, li { white-space: pre-wrap; }
      | </style></head><body style=" font-family:'Sans'; font-size:10pt; font-weight:400; font-style:normal;">
      | <p style=" margin-top:12px; margin-bottom:12px; margin-left:0px; margin-right:0px; -qt-block-indent:0; text-indent:0px;"><span style=" font-family:'MS Shell Dlg'; font-size:8pt;">o={w,x,y,z}</span></p></body></html>
  Delay : xbsIndex_r4/Delay  rst=off  infoeditControl=Selection of Reset will increase slice count due to use of real FFs and instead of SRLs  en=off  latency=7  dbl_ovrd=off  reg_retiming=off  block_type=delay
  Delay1 : xbsIndex_r4/Delay  rst=off  infoeditControl=Selection of Reset will increase slice count due to use of real FFs and instead of SRLs  en=off  latency=7  dbl_ovrd=off  reg_retiming=off  block_type=delay
  gain_and_quantize : Subsystem
  gain_and_quantize1 : Subsystem
  gain_and_quantize2 : Subsystem
  gain_and_quantize3 : Subsystem
      settings: AttributesFormatString=latency = 7
  simd_vacc : Subsystem
  sync_out : Outport
  valid : Outport  Port=2
  sum16 : Outport  Port=3
  wires:
  gain_and_quantize/1(out4) -> BitBasher2/1
  gain/1 -> gain_and_quantize/1(g), gain_and_quantize1/1(g), gain_and_quantize2/1(g), gain_and_quantize3/1(g)
  gain_and_quantize1/1(out4) -> BitBasher2/2
  simd_vacc/3(w) -> gain_and_quantize/2(in8)
  gain_and_quantize2/1(out4) -> BitBasher2/3
  simd_vacc/6(z) -> gain_and_quantize3/2(in8)
  sync_in/1 -> simd_vacc/1(sync)
  in32/1 -> simd_vacc/2(in)
  simd_vacc/4(x) -> gain_and_quantize1/2(in8)
  simd_vacc/5(y) -> gain_and_quantize2/2(in8)
  simd_vacc/1(sync_out) -> Delay/1
  simd_vacc/2(valid) -> Delay1/1
  BitBasher2/1 -> sum16/1
  gain_and_quantize3/1(out4) -> BitBasher2/4
  Delay1/1 -> valid/1
  Delay/1 -> sync_out/1
  disable/1 -> simd_vacc/3(disable)

== system sma_corr/beng/sum2/sum_Xcd ==
  gain : Inport
  sync_in : Inport  Port=2
  in32 : Inport  Port=3
  disable : Inport  Port=4
  BitBasher2 : xbsIndex_r4/BitBasher  bitexpr=|  display_expr=off  sr_1=1  arith_type1=Unsigned  bin_pt1=0  sr_2=2  arith_type2=Unsigned  bin_pt2=0  sr_3=3  arith_type3=Unsigned  bin_pt3=0  sr_4=4  arith_type4=Unsigned  bin_pt4=0  dbl_ovrd=off  block_type=bitbasher
      | <!DOCTYPE HTML PUBLIC "-//W3C//DTD HTML 4.0//EN" "http://www.w3.org/TR/REC-html40/strict.dtd">
      | <html><head><meta name="qrichtext" content="1" /><style type="text/css">
      | p, li { white-space: pre-wrap; }
      | </style></head><body style=" font-family:'Sans'; font-size:10pt; font-weight:400; font-style:normal;">
      | <p style=" margin-top:12px; margin-bottom:12px; margin-left:0px; margin-right:0px; -qt-block-indent:0; text-indent:0px;"><span style=" font-family:'MS Shell Dlg'; font-size:8pt;">o={w,x,y,z}</span></p></body></html>
  Delay : xbsIndex_r4/Delay  rst=off  infoeditControl=Selection of Reset will increase slice count due to use of real FFs and instead of SRLs  en=off  latency=7  dbl_ovrd=off  reg_retiming=off  block_type=delay
  Delay1 : xbsIndex_r4/Delay  rst=off  infoeditControl=Selection of Reset will increase slice count due to use of real FFs and instead of SRLs  en=off  latency=7  dbl_ovrd=off  reg_retiming=off  block_type=delay
  gain_and_quantize : Subsystem
  gain_and_quantize1 : Subsystem
  gain_and_quantize2 : Subsystem
  gain_and_quantize3 : Subsystem
      settings: AttributesFormatString=latency = 7
  simd_vacc : Subsystem
  sync_out : Outport
  valid : Outport  Port=2
  sum16 : Outport  Port=3
  wires:
  gain_and_quantize/1(out4) -> BitBasher2/1
  gain/1 -> gain_and_quantize/1(g), gain_and_quantize1/1(g), gain_and_quantize2/1(g), gain_and_quantize3/1(g)
  gain_and_quantize1/1(out4) -> BitBasher2/2
  simd_vacc/3(w) -> gain_and_quantize/2(in8)
  gain_and_quantize2/1(out4) -> BitBasher2/3
  simd_vacc/6(z) -> gain_and_quantize3/2(in8)
  sync_in/1 -> simd_vacc/1(sync)
  in32/1 -> simd_vacc/2(in)
  simd_vacc/4(x) -> gain_and_quantize1/2(in8)
  simd_vacc/5(y) -> gain_and_quantize2/2(in8)
  simd_vacc/1(sync_out) -> Delay/1
  simd_vacc/2(valid) -> Delay1/1
  BitBasher2/1 -> sum16/1
  gain_and_quantize3/1(out4) -> BitBasher2/4
  Delay1/1 -> valid/1
  Delay/1 -> sync_out/1
  disable/1 -> simd_vacc/3(disable)

== system sma_corr/beng/sum2/sum_Yab ==
  gain : Inport
  sync_in : Inport  Port=2
  in32 : Inport  Port=3
  disable : Inport  Port=4
  BitBasher2 : xbsIndex_r4/BitBasher  bitexpr=|  display_expr=off  sr_1=1  arith_type1=Unsigned  bin_pt1=0  sr_2=2  arith_type2=Unsigned  bin_pt2=0  sr_3=3  arith_type3=Unsigned  bin_pt3=0  sr_4=4  arith_type4=Unsigned  bin_pt4=0  dbl_ovrd=off  block_type=bitbasher
      | <!DOCTYPE HTML PUBLIC "-//W3C//DTD HTML 4.0//EN" "http://www.w3.org/TR/REC-html40/strict.dtd">
      | <html><head><meta name="qrichtext" content="1" /><style type="text/css">
      | p, li { white-space: pre-wrap; }
      | </style></head><body style=" font-family:'Sans'; font-size:10pt; font-weight:400; font-style:normal;">
      | <p style=" margin-top:12px; margin-bottom:12px; margin-left:0px; margin-right:0px; -qt-block-indent:0; text-indent:0px;"><span style=" font-family:'MS Shell Dlg'; font-size:8pt;">o={w,x,y,z}</span></p></body></html>
  Delay : xbsIndex_r4/Delay  rst=off  infoeditControl=Selection of Reset will increase slice count due to use of real FFs and instead of SRLs  en=off  latency=7  dbl_ovrd=off  reg_retiming=off  block_type=delay
  Delay1 : xbsIndex_r4/Delay  rst=off  infoeditControl=Selection of Reset will increase slice count due to use of real FFs and instead of SRLs  en=off  latency=7  dbl_ovrd=off  reg_retiming=off  block_type=delay
  gain_and_quantize : Subsystem
  gain_and_quantize1 : Subsystem
  gain_and_quantize2 : Subsystem
  gain_and_quantize3 : Subsystem
      settings: AttributesFormatString=latency = 7
  simd_vacc : Subsystem
  sync_out : Outport
  valid : Outport  Port=2
  sum16 : Outport  Port=3
  wires:
  gain_and_quantize/1(out4) -> BitBasher2/1
  gain/1 -> gain_and_quantize/1(g), gain_and_quantize1/1(g), gain_and_quantize2/1(g), gain_and_quantize3/1(g)
  gain_and_quantize1/1(out4) -> BitBasher2/2
  simd_vacc/3(w) -> gain_and_quantize/2(in8)
  gain_and_quantize2/1(out4) -> BitBasher2/3
  simd_vacc/6(z) -> gain_and_quantize3/2(in8)
  sync_in/1 -> simd_vacc/1(sync)
  in32/1 -> simd_vacc/2(in)
  simd_vacc/4(x) -> gain_and_quantize1/2(in8)
  simd_vacc/5(y) -> gain_and_quantize2/2(in8)
  simd_vacc/1(sync_out) -> Delay/1
  simd_vacc/2(valid) -> Delay1/1
  BitBasher2/1 -> sum16/1
  gain_and_quantize3/1(out4) -> BitBasher2/4
  Delay1/1 -> valid/1
  Delay/1 -> sync_out/1
  disable/1 -> simd_vacc/3(disable)

== system sma_corr/beng/sum2/sum_Ycd ==
  gain : Inport
  sync_in : Inport  Port=2
  in32 : Inport  Port=3
  disable : Inport  Port=4
  BitBasher2 : xbsIndex_r4/BitBasher  bitexpr=|  display_expr=off  sr_1=1  arith_type1=Unsigned  bin_pt1=0  sr_2=2  arith_type2=Unsigned  bin_pt2=0  sr_3=3  arith_type3=Unsigned  bin_pt3=0  sr_4=4  arith_type4=Unsigned  bin_pt4=0  dbl_ovrd=off  block_type=bitbasher
      | <!DOCTYPE HTML PUBLIC "-//W3C//DTD HTML 4.0//EN" "http://www.w3.org/TR/REC-html40/strict.dtd">
      | <html><head><meta name="qrichtext" content="1" /><style type="text/css">
      | p, li { white-space: pre-wrap; }
      | </style></head><body style=" font-family:'Sans'; font-size:10pt; font-weight:400; font-style:normal;">
      | <p style=" margin-top:12px; margin-bottom:12px; margin-left:0px; margin-right:0px; -qt-block-indent:0; text-indent:0px;"><span style=" font-family:'MS Shell Dlg'; font-size:8pt;">o={w,x,y,z}</span></p></body></html>
  Delay : xbsIndex_r4/Delay  rst=off  infoeditControl=Selection of Reset will increase slice count due to use of real FFs and instead of SRLs  en=off  latency=7  dbl_ovrd=off  reg_retiming=off  block_type=delay
  Delay1 : xbsIndex_r4/Delay  rst=off  infoeditControl=Selection of Reset will increase slice count due to use of real FFs and instead of SRLs  en=off  latency=7  dbl_ovrd=off  reg_retiming=off  block_type=delay
  gain_and_quantize : Subsystem
  gain_and_quantize1 : Subsystem
  gain_and_quantize2 : Subsystem
  gain_and_quantize3 : Subsystem
      settings: AttributesFormatString=latency = 7
  simd_vacc : Subsystem
  sync_out : Outport
  valid : Outport  Port=2
  sum16 : Outport  Port=3
  wires:
  gain_and_quantize/1(out4) -> BitBasher2/1
  gain/1 -> gain_and_quantize/1(g), gain_and_quantize1/1(g), gain_and_quantize2/1(g), gain_and_quantize3/1(g)
  gain_and_quantize1/1(out4) -> BitBasher2/2
  simd_vacc/3(w) -> gain_and_quantize/2(in8)
  gain_and_quantize2/1(out4) -> BitBasher2/3
  simd_vacc/6(z) -> gain_and_quantize3/2(in8)
  sync_in/1 -> simd_vacc/1(sync)
  in32/1 -> simd_vacc/2(in)
  simd_vacc/4(x) -> gain_and_quantize1/2(in8)
  simd_vacc/5(y) -> gain_and_quantize2/2(in8)
  simd_vacc/1(sync_out) -> Delay/1
  simd_vacc/2(valid) -> Delay1/1
  BitBasher2/1 -> sum16/1
  gain_and_quantize3/1(out4) -> BitBasher2/4
  Delay1/1 -> valid/1
  Delay/1 -> sync_out/1
  disable/1 -> simd_vacc/3(disable)

== system sma_corr/beng/sum3/sum_Xab ==
  gain : Inport
  sync_in : Inport  Port=2
  in32 : Inport  Port=3
  disable : Inport  Port=4
  BitBasher2 : xbsIndex_r4/BitBasher  bitexpr=|  display_expr=off  sr_1=1  arith_type1=Unsigned  bin_pt1=0  sr_2=2  arith_type2=Unsigned  bin_pt2=0  sr_3=3  arith_type3=Unsigned  bin_pt3=0  sr_4=4  arith_type4=Unsigned  bin_pt4=0  dbl_ovrd=off  block_type=bitbasher
      | <!DOCTYPE HTML PUBLIC "-//W3C//DTD HTML 4.0//EN" "http://www.w3.org/TR/REC-html40/strict.dtd">
      | <html><head><meta name="qrichtext" content="1" /><style type="text/css">
      | p, li { white-space: pre-wrap; }
      | </style></head><body style=" font-family:'Sans'; font-size:10pt; font-weight:400; font-style:normal;">
      | <p style=" margin-top:12px; margin-bottom:12px; margin-left:0px; margin-right:0px; -qt-block-indent:0; text-indent:0px;"><span style=" font-family:'MS Shell Dlg'; font-size:8pt;">o={w,x,y,z}</span></p></body></html>
  Delay : xbsIndex_r4/Delay  rst=off  infoeditControl=Selection of Reset will increase slice count due to use of real FFs and instead of SRLs  en=off  latency=7  dbl_ovrd=off  reg_retiming=off  block_type=delay
  Delay1 : xbsIndex_r4/Delay  rst=off  infoeditControl=Selection of Reset will increase slice count due to use of real FFs and instead of SRLs  en=off  latency=7  dbl_ovrd=off  reg_retiming=off  block_type=delay
  gain_and_quantize : Subsystem
  gain_and_quantize1 : Subsystem
  gain_and_quantize2 : Subsystem
  gain_and_quantize3 : Subsystem
      settings: AttributesFormatString=latency = 7
  simd_vacc : Subsystem
  sync_out : Outport
  valid : Outport  Port=2
  sum16 : Outport  Port=3
  wires:
  gain_and_quantize/1(out4) -> BitBasher2/1
  gain/1 -> gain_and_quantize/1(g), gain_and_quantize1/1(g), gain_and_quantize2/1(g), gain_and_quantize3/1(g)
  gain_and_quantize1/1(out4) -> BitBasher2/2
  simd_vacc/3(w) -> gain_and_quantize/2(in8)
  gain_and_quantize2/1(out4) -> BitBasher2/3
  simd_vacc/6(z) -> gain_and_quantize3/2(in8)
  sync_in/1 -> simd_vacc/1(sync)
  in32/1 -> simd_vacc/2(in)
  simd_vacc/4(x) -> gain_and_quantize1/2(in8)
  simd_vacc/5(y) -> gain_and_quantize2/2(in8)
  simd_vacc/1(sync_out) -> Delay/1
  simd_vacc/2(valid) -> Delay1/1
  BitBasher2/1 -> sum16/1
  gain_and_quantize3/1(out4) -> BitBasher2/4
  Delay1/1 -> valid/1
  Delay/1 -> sync_out/1
  disable/1 -> simd_vacc/3(disable)
